simulink model "modelo_naolinear"  (R2023a)
settings: Solver=ode4  StartTime=0.0  StopTime=T_sim  FixedStep=dt  RelTol=1e-3  EnableMultiTasking=on
InitFcn:
  | modelo_naolinear_params
values from neighbouring files (symbols this model uses):
  B22 = B(7:12,2:4)    [modelo_naolinear_params.m]
  dt = 1e-3    (= 0.001)    [modelo_naolinear_params.m]
  G = inv(K*B22*inv(R)*B22'-A22')*C'*Q    [modelo_naolinear_params.m]
  g = 9.8    [modelo_naolinear_params.m]
  incluir_din_motor = 1    [modelo_naolinear_params.m]
  input_selector = 1    [modelo_naolinear_params.m]
  Ir = 1e-3    (= 0.001)    [modelo_naolinear_params.m]
  Ixx = 1e-2    (= 0.01)    [modelo_naolinear_params.m]
  Iyy = 1e-2    (= 0.01)    [modelo_naolinear_params.m]
  Izz = 1.8e-2    (= 0.018)    [modelo_naolinear_params.m]
  K = are(A22, B22*inv(R)*B22',C'*Q*C)    [modelo_naolinear_params.m]
  Kd_rot = 0.01    [modelo_naolinear_params.m]
  Kd_trans = -0.13723    [modelo_naolinear_params.m]
  Ki_rot = 1.0    (= 1)    [modelo_naolinear_params.m]
  Ki_trans = 0.4    [modelo_naolinear_params.m]
  Kp = 0.9182    [modelo_naolinear_params.m]
  Kp_rot = 10    [modelo_naolinear_params.m]
  Kp_trans = 2.0968    [modelo_naolinear_params.m]
  m = 1.0428    [modelo_naolinear_params.m]
  N_rot = 100    [modelo_naolinear_params.m]
  N_trans = 5.5815    [modelo_naolinear_params.m]
  p0 = 0    [modelo_naolinear_params.m]
  phi0 = 0    [modelo_naolinear_params.m]
  psi0 = 0    [modelo_naolinear_params.m]
  q0 = 0    [modelo_naolinear_params.m]
  R = diag([r11,r22,r33])    [modelo_naolinear_params.m]
  r0 = 0    [modelo_naolinear_params.m]
  seleciona_controlador = -1    [modelo_naolinear_params.m]
  T = [kf,kf,kf,kf ... (first row only)    [modelo_naolinear_params.m]
  T_inv = T^(-1)    [modelo_naolinear_params.m]
  tau_a = 0.0569    [modelo_naolinear_params.m]
  tau_s = 0.045    [modelo_naolinear_params.m]
  theta0 = 0    [modelo_naolinear_params.m]
  vx0 = 0    [modelo_naolinear_params.m]
  vy0 = 0    [modelo_naolinear_params.m]
  vz0 = 0    [modelo_naolinear_params.m]
  x0 = 0    [modelo_naolinear_params.m]
  y0 = 0    [modelo_naolinear_params.m]
  z0 = 1    [modelo_naolinear_params.m]
undefined symbols (not defined in the file or in its folder): sigbuilder_block
libraries: slpidlib x6, aerolibanimutils x2, sim3dlib x1, uavsim3dlib x1
(part 1 of 2)
settings Model: Creator=dferr  LastModifiedBy=dferr
settings SolverCC: AlgebraicLoopSolver=TrustRegion  AutoInsertRateTranBlk=on
settings DataIOCC: LimitDataPoints=on  SaveFormat=Array  ReturnWorkspaceOutputs=off
settings OptimizationCC: LifeSpan=inf
settings DebuggingCC: UnconnectedInputMsg=warning  UnconnectedOutputMsg=warning  UnconnectedLineMsg=warning  SFUnexpectedBacktrackingDiag=warning  SFNoUnconditionalDefaultTransitionDiag=warning  SFExecutionAtInitializationDiag=none  ArithmeticOperatorsInVariantConditions=warning
settings HardwareCC: ProdBitPerPointer=32  ProdBitPerSizeT=32  ProdBitPerPtrDiffT=32  ProdLargestAtomicFloat=None  ProdIntDivRoundTo=Undefined  ProdEndianess=Unspecified  ProdWordSize=32  ProdHWDeviceType=32-bit Generic
settings ModelReferenceCC: EnableRefExpFcnMdlSchedulingChecks=on  PropagateSignalLabelsOutOfModel=off
settings SFSimCC: SimUseLocalCustomCode=off  CustomCodeUndefinedFunction=UseInterfaceOnly
settings RTWCC: RTWUseLocalCustomCode=off
settings CodeAppCC: StateflowObjectComments=on
settings GRTTargetCC: TargetLangStandard=C89/C90 (ANSI)
settings ConfigComp: CovMetricSettings=dw  CovSaveCumulativeToWorkspaceVar=on  CovSaveSingleToWorkspaceVar=on  CovExternalEMLEnable=off  CovSFcnEnable=off

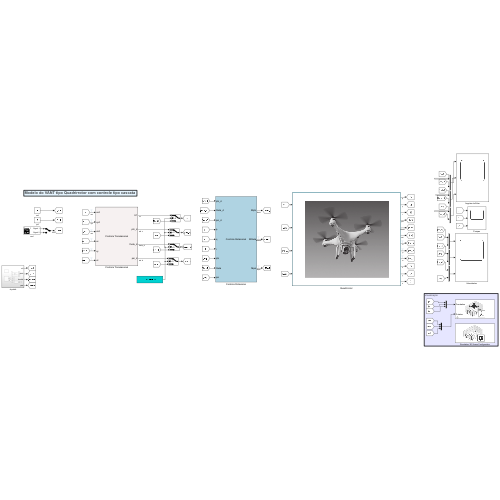
[thumbnail: Simulink's stored preview of the top-level diagram]
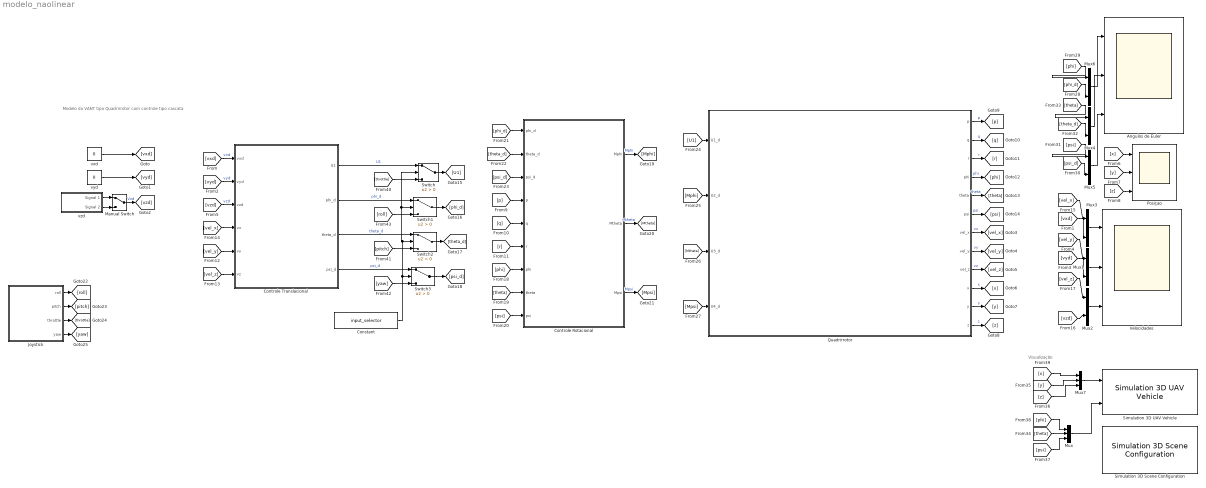
== system modelo_naolinear ==
  note: Visualização
  note: Modelo do VANT tipo Quadrirrotor com controle tipo cascata
  Angulos de Euler : Scope  NumInputPorts=3
  Constant : Constant  Value=input_selector
  Controle Rotacional : Subsystem
      mask Display:
      | disp('Controle Rotacional')
      | port_label('input',1,'phi_d')
      | port_label('input',2,'theta_d')
      | port_label('input',3,'psi_d')
      | port_label('input',4,'p')
      | port_label('input',5,'q')
      | port_label('input',6,'r')
      | port_label('input',7,'phi')
      | port_label('input',8,'theta')
      | port_label('input',9,'psi')
      | port_label('output',1,'Mphi')
      | port_label('output',2,'Mtheta')
      | port_label('output',3,'Mpsi')
  Controle Translacional : Subsystem
      mask Display:
      | disp('Controle Translacional')
      | port_label('input',1,'vxd')
      | port_label('input',2,'vyd')
      | port_label('input',3,'vzd')
      | port_label('input',4,'vx')
      | port_label('input',5,'vy')
      | port_label('input',6,'vz')
      | port_label('output',1,'U1')
      | port_label('output',2,'phi_d')
      | port_label('output',3,'theta_d')
      | port_label('output',4,'psi_d')
  Joystick : Subsystem
  Manual Switch : ManualSwitch
  Mux : Mux  Inputs=3
  Mux{1..6} (6 alike) : Mux  Inputs=2
  Mux7 : Mux  Inputs=3
  Posiçao : Scope  NumInputPorts=3
  Quadrirrotor : Subsystem
      mask init:
      | x = imread('figures/drone.png');
      | set_param(gcb,'UserDataPersistent','on');
      | ud.x = x;
      | set_param(gcb,'UserData',ud);
      mask Display:
      | disp('Quadrirrotor')
      | port_label('input',1,'U1')
      | port_label('input',2,'U2')
      | port_label('input',3,'U3')
      | port_label('input',4,'U4')
      | port_label('output',1,'p')
      | port_label('output',2,'q')
      | port_label('output',3,'r')
      | port_label('output',4,'phi')
      | port_label('output',5,'theta')
      | port_label('output',6,'psi')
      | port_label('output',7,'vel_x')
      | port_label('output',8,'vel_y')
      | port_label('output',9,'vel_z')
      | port_label('output',10,'x')
      | port_label('output',11,'y')
      | port_label('output',12,'z')
      | ud = get_param(gcb,'UserData')
      | image(ud.x)
  Simulation 3D Scene Configuration : sim3dlib/Simulation 3D Scene Configuration  Priority=0  ProjectFormat=Default Scenes  SceneDesc=US city block  ProjectName=C:\Program Files\MATLAB\R2023a\toolbox\shared\sim3d_projects\automotive_project\UE4\WindowsNoEditor\VehicleSimulation.exe  ScenePath=/Game/Maps/USCityBlock  EnableOpenDRIVE=off  vehTagList={'Scene Origin';'SimulinkVehicle1'}  vehTag=SimulinkVehicle1  OpVisEn=off  Ts=0.01  EnableWindow=on  EnableRemoteAccess=off  EnableWeather=off  SunAltitude=40.0  SunAzimuth=90  clouds=10  wind=1  fog=0  rain=0  EnableGeospatial=off  OriginHeight=200  OriginLatitude=40.744652  OriginLongitude=-73.988864  MapStyle=Aerial  AdditionalAssetIDs=[]  UseAdvancedSunSky=off  SolarTime=11  TimeZone=-5  Day=21  Month=9  Year=2022  UseDaylightSavingTime=on  DSTStartDay=10  DSTStartMonth=3  DSTEndDay=3  DSTEndMonth=11  DSTSwitchHour=2  sensorTag=MainCamera1  sensorId=1  sensorName=MainCamera1  mountLoc=Origin  offsetFlag=off  tmountOffset=[0, 0, 0]  rmountOffset=[0, 0, 0]  HorizontalResolution=1280  VerticalResolution=720  HorizontalFOV=60  mountPoint=[0 0 0]  mountOrientation=[0 0 0]  aMode=4  ID=1  GeoTrigger=Inactive
      settings: LibrarySourceBlock=uavsim3dlib/Simulation 3D Scene Configuration
  Simulation 3D UAV Vehicle : uavsim3dlib/Simulation 3D UAV Vehicle  Priority=-1  Mesh=Quadrotor  MeshPath=/MathWorksUAVContent/UAVs/HexaRotorUAV/HexaRotor.HexaRotor  Color=Black  ActorName=SimulinkVehicle1  UseGlobalFrame=off  InitialTranslation=[0 0 0]  InitialRotation=[0 0 0]  InitialTranslationGlobal=[0 0 0]  InitialGeoOrigin=[0 0 0]  InitialRotationGlobal=[0 0 0]  SampleTime=0.01  ActorType=SimulinkVehicle  ID=1  aMode=4  ActorTag=SimulinkVehicle1
  Switch{,1..3} (4 alike) : Switch  Criteria=u2 > Threshold  SaturateOnIntegerOverflow=off  InputSameDT=off
  Velocidades : Scope  NumInputPorts=3
  vxd : Constant  Value=0
  vyd : Constant  Value=0
  vzd : Subsystem  Tag=STV Subsys  LoadFcn=sigbuilder_block('load',[1045.5 36.75 386.25 279 ]);  StartFcn=sigbuilder_block('start');  StopFcn=sigbuilder_block('stop');  ClipboardFcn=sigbuilder_block('clipboard');  mask(Sigbuilder block){tuvar=[0:10]}
      mask help: Simulink:masks:SigbuilderBlock_MD
      mask init:
      | if ~strcmp(get_param(bdroot(gcbh),'SimulationStatus'),'stopped') tuvar = sigbuilder_block('maskInit'); end
      settings: CopyFcn=sigbuilder_block('copy');  DeleteFcn=sigbuilder_block('delete');  ModelCloseFcn=sigbuilder_block('modelClose');  PreSaveFcn=sigbuilder_block('preSave');  PauseFcn=sigbuilder_block('pause');  ContinueFcn=sigbuilder_block('continue');  NameChangeFcn=sigbuilder_block('namechange');  OpenFcn=sigbuilder_block('open',[437.6 199.2 550.4 400 ]);  CloseFcn=sigbuilder_block('close');
      mask Display: plot(0, 0, 100, 100,[2, 2, 32, 32, 2], [68, 8, 8, 68, 68],[32, 2], [38, 38], [32, 19, 2],[53, 60, 44], [32, 17, 17, 2],[16, 16, 31, 31]);txt = getActiveGroup(gcbh);text(2, 100, txt,'verticalAlignment', 'top');
  wires:
  Mux3/1 -> Velocidades/1
  vzd/1(Signal 1) -> Manual Switch/1
  vzd/2(Signal 2) -> Manual Switch/2
  vxd/1 -> [vxd]
  [vxd] -> Controle Translacional/1(vxd)  "vxd"
  [vxd] -> Mux3/2
  vyd/1 -> [vyd]
  [vyd] -> Controle Translacional/2(vyd)  "vyd"
  Manual Switch/1 -> [vzd]  "Vzd"
  [vzd] -> Controle Translacional/3(vzd)  "vzd"
  [x] -> Posiçao/1
  [y] -> Posiçao/2
  [z] -> Posiçao/3
  [vel_x] -> Controle Translacional/4(vx)
  [vel_y] -> Controle Translacional/5(vy)
  [vel_z] -> Controle Translacional/6(vz)
  [vel_x] -> Mux3/1
  [vel_y] -> Mux1/1
  [vyd] -> Mux1/2
  Mux1/1 -> Velocidades/2
  Mux2/1 -> Velocidades/3
  [vzd] -> Mux2/2
  [vel_z] -> Mux2/1
  Quadrirrotor/7(vel_x) -> [vel_x]  "vx"
  Quadrirrotor/8(vel_y) -> [vel_y]  "vy"
  Quadrirrotor/9(vel_z) -> [vel_z]  "vz"
  Quadrirrotor/10(x) -> [x]  "x"
  Quadrirrotor/11(y) -> [y]  "y"
  Quadrirrotor/12(z) -> [z]  "z"
  Quadrirrotor/1(p) -> [p]  "p"
  Quadrirrotor/3(r) -> [r]  "r"
  Quadrirrotor/4(phi) -> [phi]  "phi"
  Quadrirrotor/5(theta) -> [theta]  "theta"
  Quadrirrotor/6(psi) -> [psi]  "psi"
  [p] -> Controle Rotacional/4(p)
  [q] -> Controle Rotacional/5(q)
  [r] -> Controle Rotacional/6(r)
  [phi] -> Controle Rotacional/7(phi)
  [theta] -> Controle Rotacional/8(theta)
  [psi] -> Controle Rotacional/9(psi)
  [phi_d] -> Controle Rotacional/1(phi_d)
  [theta_d] -> Controle Rotacional/2(theta_d)
  [psi_d] -> Controle Rotacional/3(psi_d)
  [U1] -> Quadrirrotor/1(U1_d)
  Controle Rotacional/1(Mphi) -> [Mphi]  "Mphi"
  Controle Rotacional/2(Mtheta) -> [Mtheta]  "Mtheta"
  Controle Rotacional/3(Mpsi) -> [Mpsi]  "Mpsi"
  [Mpsi] -> Quadrirrotor/4(U4_d)
  [psi] -> Mux5/1
  [psi_d] -> Mux5/2
  Mux5/1 -> Angulos de Euler/3
  Mux4/1 -> Angulos de Euler/2
  [theta_d] -> Mux4/2
  [theta] -> Mux4/1
  [phi] -> Mux6/1
  [phi_d] -> Mux6/2
  Mux6/1 -> Angulos de Euler/1
  [Mphi] -> Quadrirrotor/2(U2_d)
  [Mtheta] -> Quadrirrotor/3(U3_d)
  Quadrirrotor/2(q) -> [q]  "q"
  Joystick/1(roll) -> [roll]
  Joystick/2(pitch) -> [pitch]
  Joystick/3(throttle) -> [throttle]
  Joystick/4(yaw) -> [yaw]
  Switch/1 -> [U1]
  Switch1/1 -> [phi_d]
  Switch2/1 -> [theta_d]
  Switch3/1 -> [psi_d]
  Controle Translacional/1(U1) -> Switch/1  "U1"
  Controle Translacional/2(phi_d) -> Switch1/1  "phi_d"
  Controle Translacional/3(theta_d) -> Switch2/1  "theta_d"
  Controle Translacional/4(psi_d) -> Switch3/1  "psi_d"
  [throttle] -> Switch/3
  [pitch] -> Switch2/3
  [yaw] -> Switch3/3
  [roll] -> Switch1/3
  Constant/1 -> Switch3/2, Switch2/2, Switch/2, Switch1/2
  [z] -> Mux7/3
  [x] -> Mux7/1
  [psi] -> Mux/3
  [y] -> Mux7/2
  Mux/1 -> Simulation 3D UAV Vehicle/2
  [phi] -> Mux/1
  [theta] -> Mux/2
  Mux7/1 -> Simulation 3D UAV Vehicle/1

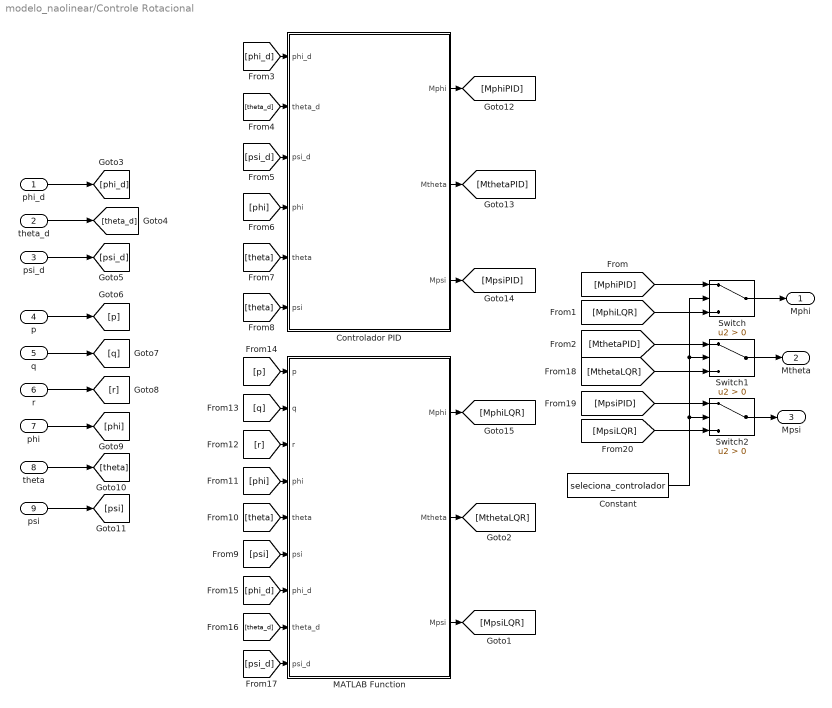
== system modelo_naolinear/Controle Rotacional ==
  phi_d : Inport
  theta_d : Inport  Port=2
  psi_d : Inport  Port=3
  p : Inport  Port=4
  q : Inport  Port=5
  r : Inport  Port=6
  phi : Inport  Port=7
  theta : Inport  Port=8
  psi : Inport  Port=9
  Constant : Constant  Value=seleciona_controlador
  Controlador PID : Subsystem
      mask Display:
      | disp('Controlador PID')
      | port_label('input',1,'phi_d')
      | port_label('input',2,'theta_d')
      | port_label('input',3,'psi_d')
      | port_label('input',4,'phi')
      | port_label('input',5,'theta')
      | port_label('input',6,'psi')
      | port_label('output',1,'Mphi')
      | port_label('output',2,'Mtheta')
      | port_label('output',3,'Mpsi')
  MATLAB Function : MATLAB Function  TreatAsAtomicUnit=on
  Switch : Switch  Criteria=u2 > Threshold  SaturateOnIntegerOverflow=off  InputSameDT=off
  Switch1 : Switch  Criteria=u2 > Threshold  SaturateOnIntegerOverflow=off  InputSameDT=off
  Switch2 : Switch  Criteria=u2 > Threshold  SaturateOnIntegerOverflow=off  InputSameDT=off
  Mphi : Outport  VectorParamsAs1DForOutWhenUnconnected=off
  Mtheta : Outport  Port=2  VectorParamsAs1DForOutWhenUnconnected=off
  Mpsi : Outport  Port=3  VectorParamsAs1DForOutWhenUnconnected=off
  wires:
  Switch/1 -> Mphi/1
  Switch1/1 -> Mtheta/1
  Switch2/1 -> Mpsi/1
  phi_d/1 -> [phi_d]
  theta_d/1 -> [theta_d]
  psi_d/1 -> [psi_d]
  p/1 -> [p]
  q/1 -> [q]
  r/1 -> [r]
  phi/1 -> [phi]
  theta/1 -> [theta]
  psi/1 -> [psi]
  [phi_d] -> Controlador PID/1(phi_d)
  [theta_d] -> Controlador PID/2(theta_d)
  [psi_d] -> Controlador PID/3(psi_d)
  [phi] -> Controlador PID/4(phi)
  [theta] -> Controlador PID/5(theta)
  [theta] -> Controlador PID/6(psi)
  Controlador PID/3(Mpsi) -> [MpsiPID]
  Controlador PID/2(Mtheta) -> [MthetaPID]
  Controlador PID/1(Mphi) -> [MphiPID]
  [psi] -> MATLAB Function/6(psi)
  [theta] -> MATLAB Function/5(theta)
  [phi] -> MATLAB Function/4(phi)
  [r] -> MATLAB Function/3(r)
  [q] -> MATLAB Function/2(q)
  [p] -> MATLAB Function/1(p)
  [phi_d] -> MATLAB Function/7(phi_d)
  [theta_d] -> MATLAB Function/8(theta_d)
  [psi_d] -> MATLAB Function/9(psi_d)
  MATLAB Function/1(Mphi) -> [MphiLQR]
  MATLAB Function/2(Mtheta) -> [MthetaLQR]
  MATLAB Function/3(Mpsi) -> [MpsiLQR]
  [MphiPID] -> Switch/1
  [MphiLQR] -> Switch/3
  [MthetaPID] -> Switch1/1
  [MthetaLQR] -> Switch1/3
  [MpsiPID] -> Switch2/1
  [MpsiLQR] -> Switch2/3
  Constant/1 -> Switch2/2, Switch1/2, Switch/2

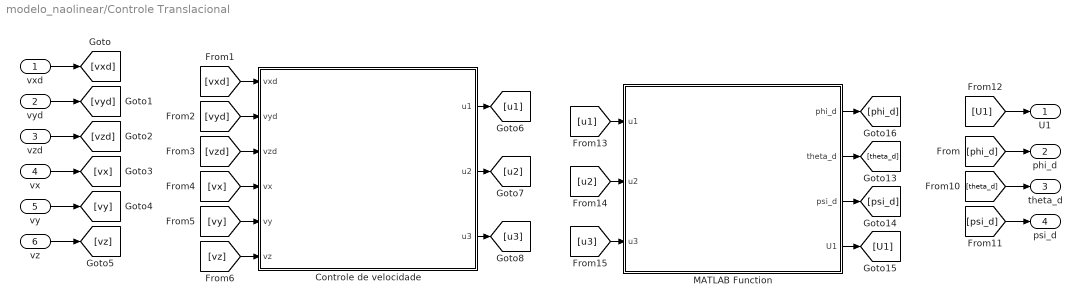
== system modelo_naolinear/Controle Translacional ==
  vxd : Inport
  vyd : Inport  Port=2
  vzd : Inport  Port=3
  vx : Inport  Port=4
  vy : Inport  Port=5
  vz : Inport  Port=6
  Controle de velocidade : Subsystem
      mask Display:
      | disp('Controle de Velocidade PID')
      | port_label('input',1,'vxd')
      | port_label('input',2,'vyd')
      | port_label('input',3,'vzd')
      | port_label('input',4,'vx')
      | port_label('input',5,'vy')
      | port_label('input',6,'vz')
      | 
      | port_label('output',1,'u1')
      | port_label('output',2,'u2')
      | port_label('output',3,'u3')
  MATLAB Function : MATLAB Function  TreatAsAtomicUnit=on
  U1 : Outport  VectorParamsAs1DForOutWhenUnconnected=off
  phi_d : Outport  Port=2  VectorParamsAs1DForOutWhenUnconnected=off
  theta_d : Outport  Port=3  VectorParamsAs1DForOutWhenUnconnected=off
  psi_d : Outport  Port=4  VectorParamsAs1DForOutWhenUnconnected=off
  wires:
  vxd/1 -> [vxd]
  vyd/1 -> [vyd]
  vzd/1 -> [vzd]
  vx/1 -> [vx]
  vy/1 -> [vy]
  vz/1 -> [vz]
  [vxd] -> Controle de velocidade/1(vxd)
  [vyd] -> Controle de velocidade/2(vyd)
  [vzd] -> Controle de velocidade/3(vzd)
  [vx] -> Controle de velocidade/4(vx)
  [vy] -> Controle de velocidade/5("vy ")
  [vz] -> Controle de velocidade/6(vz)
  Controle de velocidade/1(u1) -> [u1]
  Controle de velocidade/2(u2) -> [u2]
  Controle de velocidade/3(u3) -> [u3]
  [phi_d] -> phi_d/1
  [theta_d] -> theta_d/1
  [psi_d] -> psi_d/1
  [U1] -> U1/1
  [u1] -> MATLAB Function/1(u1)
  [u2] -> MATLAB Function/2(u2)
  [u3] -> MATLAB Function/3(u3)
  MATLAB Function/1(phi_d) -> [phi_d]
  MATLAB Function/2(theta_d) -> [theta_d]
  MATLAB Function/3(psi_d) -> [psi_d]
  MATLAB Function/4(U1) -> [U1]

== system modelo_naolinear/Joystick ==
  Demux : Demux  Outputs=6
  Fcn : Fcn  Expr=(u(1)-0.5)*2
  Gain : Gain  Gain=-1
  Pilot Joystick1 : aerolibanimutils/Pilot Joystick  JoystickID=None  OutputConfiguration=AllOutputs  SampleTime=dt
  Simulation Pace : aerolibanimutils/Simulation Pace  SimulationPace=1  SleepMode=Auto  OutputPaceError=off  SampleTime=dt
  Terminator{,1..3} (4 alike) : Terminator
  roll : Outport
  pitch : Outport  Port=2
  throttle : Outport  Port=3
  yaw : Outport  Port=4
  wires:
  Demux/4 -> Terminator2/1
  Gain/1 -> pitch/1  "pitch"
  Pilot Joystick1/3 -> Terminator1/1
  Pilot Joystick1/1 -> Demux/1
  Demux/6 -> Fcn/1
  Pilot Joystick1/2 -> Terminator/1
  Demux/2 -> Gain/1
  Demux/1 -> roll/1  "roll"
  Demux/5 -> Terminator3/1
  Demux/3 -> throttle/1  "throttle"
  Fcn/1 -> yaw/1  "yaw"

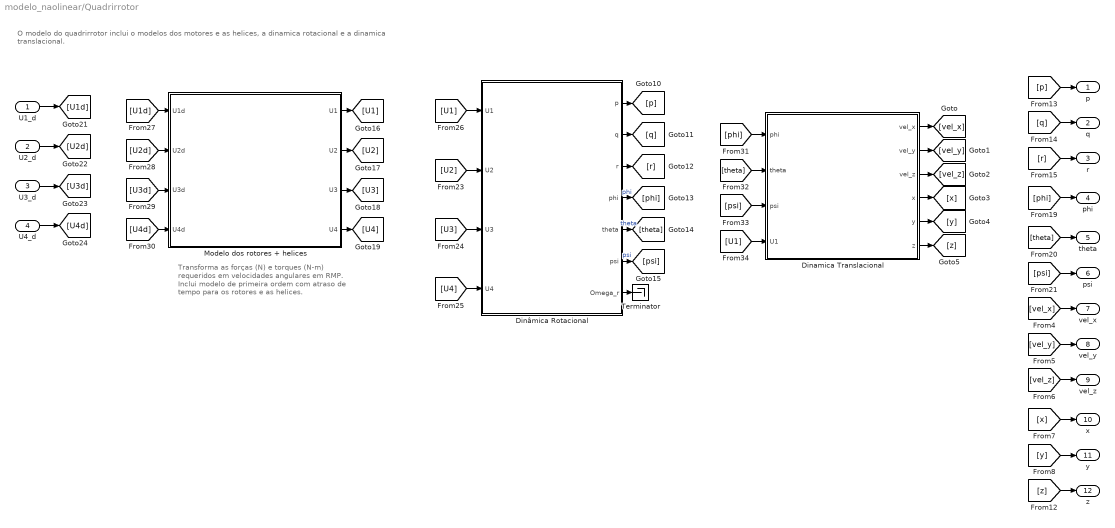
== system modelo_naolinear/Quadrirrotor ==
  note: O modelo do quadrirrotor inclui o modelos dos motores e as helices,
      | a dinamica rotacional e a dinamica translacional.
  note: Transforma as forças (N) e torques (N-m)
      | requeridos em velocidades angulares
      | em RMP. Inclui modelo de primeira
      | ordem com atraso de tempo para
      | os rotores e as helices.
  U1_d : Inport
  U2_d : Inport  Port=2
  U3_d : Inport  Port=3
  U4_d : Inport  Port=4
  Dinamica Translacional : Subsystem
  Dinâmica Rotacional : Subsystem
      mask Display:
      | disp('Dinâmica Rotacional')
      | port_label('input',1,'U1')
      | port_label('input',2,'U2')
      | port_label('input',3,'U3')
      | port_label('input',4,'U4')
      | port_label('output',1,'p')
      | port_label('output',2,'q')
      | port_label('output',3,'r')
      | port_label('output',4,'phi')
      | port_label('output',5,'theta')
      | port_label('output',6,'psi')
      | port_label('output',7,'Omega_r')
  "Modelo dos rotores + helices " : Subsystem
      mask init:
      | x = imread('figures/brushless_propellers.png');
      | set_param(gcb,'UserDataPersistent','on');
      | ud.x = x;
      | set_param(gcb,'UserData',ud);
      mask Display:
      | disp('Dinâmica Motores')
      | port_label('input',1,'U1d')
      | port_label('input',2,'U2d')
      | port_label('input',3,'U3d')
      | port_label('input',4,'U4d')
      | port_label('output',1,'U1')
      | port_label('output',2,'U2')
      | port_label('output',3,'U3')
      | port_label('output',4,'U4')
      | image(ud.x)
  Terminator : Terminator
  p : Outport  VectorParamsAs1DForOutWhenUnconnected=off
  q : Outport  Port=2  VectorParamsAs1DForOutWhenUnconnected=off
  r : Outport  Port=3  VectorParamsAs1DForOutWhenUnconnected=off
  phi : Outport  Port=4  VectorParamsAs1DForOutWhenUnconnected=off
  theta : Outport  Port=5  VectorParamsAs1DForOutWhenUnconnected=off
  psi : Outport  Port=6  VectorParamsAs1DForOutWhenUnconnected=off
  vel_x : Outport  Port=7  VectorParamsAs1DForOutWhenUnconnected=off
  vel_y : Outport  Port=8  VectorParamsAs1DForOutWhenUnconnected=off
  vel_z : Outport  Port=9  VectorParamsAs1DForOutWhenUnconnected=off
  x : Outport  Port=10  VectorParamsAs1DForOutWhenUnconnected=off
  y : Outport  Port=11  VectorParamsAs1DForOutWhenUnconnected=off
  z : Outport  Port=12  VectorParamsAs1DForOutWhenUnconnected=off
  wires:
  [vel_x] -> vel_x/1
  [vel_y] -> vel_y/1
  [vel_z] -> vel_z/1
  [z] -> z/1
  [y] -> y/1
  [x] -> x/1
  Dinâmica Rotacional/3(r) -> [r]
  Dinâmica Rotacional/2(q) -> [q]
  Dinâmica Rotacional/1(p) -> [p]
  [p] -> p/1
  [q] -> q/1
  [r] -> r/1
  Dinâmica Rotacional/5(theta) -> [theta]  "theta"
  Dinâmica Rotacional/6(psi) -> [psi]  "psi"
  [phi] -> phi/1
  [theta] -> theta/1
  [psi] -> psi/1
  "Modelo dos rotores + helices "/1(U1) -> [U1]
  "Modelo dos rotores + helices "/2(U2) -> [U2]
  "Modelo dos rotores + helices "/3(U3) -> [U3]
  "Modelo dos rotores + helices "/4(U4) -> [U4]
  [U1d] -> "Modelo dos rotores + helices "/1(U1d)
  [U2d] -> "Modelo dos rotores + helices "/2(U2d)
  [U3d] -> "Modelo dos rotores + helices "/3(U3d)
  [U4d] -> "Modelo dos rotores + helices "/4(U4d)
  U1_d/1 -> [U1d]
  U2_d/1 -> [U2d]
  U3_d/1 -> [U3d]
  U4_d/1 -> [U4d]
  [U4] -> Dinâmica Rotacional/4(U4)
  [U3] -> Dinâmica Rotacional/3(U3)
  [U2] -> Dinâmica Rotacional/2(U2)
  [U1] -> Dinâmica Rotacional/1(U1)
  Dinâmica Rotacional/7(Omega_r) -> Terminator/1
  Dinâmica Rotacional/4(phi) -> [phi]  "phi"
  [phi] -> Dinamica Translacional/1(phi)
  [theta] -> Dinamica Translacional/2(theta)
  [psi] -> Dinamica Translacional/3(psi)
  [U1] -> Dinamica Translacional/4(U1)
  Dinamica Translacional/1(vel_x) -> [vel_x]
  Dinamica Translacional/2(vel_y) -> [vel_y]
  Dinamica Translacional/3(vel_z) -> [vel_z]
  Dinamica Translacional/4(x) -> [x]
  Dinamica Translacional/5(y) -> [y]
  Dinamica Translacional/6(z) -> [z]

== system modelo_naolinear/vzd ==
  Demux : Demux  Tag=STV Demux  Outputs=2
      settings: DisplayOption=none
  FromWs : FromWorkspace  Tag=STV FromWs  VariableName=tuvar
      SigBuilderData=|
      | common.afterFinalStr = 'Extrapolate'
      | common.sampleTime = 0
      | dataSet.timeRange = [0 20]
      | dataSet.name = 'Group 1'
      | sbobj.Groups.Name = 'Group 1'
      | sbobj.Groups.Signals(1).Name = 'Signal 1'
      | sbobj.Groups.Signals(1).XData = [0 2.05 4.75 8 18 20]
      | sbobj.Groups.Signals(1).YData = [-0.2 4.2 10 10 0 0]
      | sbobj.Groups.Signals(2).Name = 'Signal 2'
      | sbobj.Groups.Signals(2).XData = [0 5 5 20]
      | sbobj.Groups.Signals(2).YData = [0 0 1 1]
      | sbobj.Groups.TimeRange = [0 20]
  Signal 1 : Outport  Tag=STV Outport  VectorParamsAs1DForOutWhenUnconnected=off
  Signal 2 : Outport  Tag=STV Outport  Port=2  VectorParamsAs1DForOutWhenUnconnected=off
  wires:
  FromWs/1 -> Demux/1
  Demux/1 -> Signal 1/1
  Demux/2 -> Signal 2/1

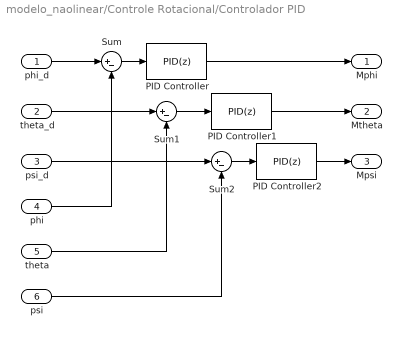
== system modelo_naolinear/Controle Rotacional/Controlador PID ==
  phi_d : Inport
  theta_d : Inport  Port=2
  psi_d : Inport  Port=3
  phi : Inport  Port=4
  theta : Inport  Port=5
  psi : Inport  Port=6
  PID Controller : slpidlib/PID Controller  Controller=PID  Form=Parallel  TimeDomain=Discrete-time  UseExternalTs=off  SampleTime=-1  IntegratorMethod=Forward Euler  FilterMethod=Forward Euler  ControllerParametersSource=internal  P=Kp_rot  I=Ki_rot  UseKiTs=off  D=Kd_rot  N=N_rot  UseFilter=on  TunerSelectOption=Transfer Function Based (PID Tuner App)  InitialConditionSource=internal  InitialConditionForIntegrator=0  InitialConditionForFilter=0  DifferentiatorICPrevScaledInput=0  ExternalReset=none  TrackingMode=off  Kt=1  LimitOutput=on  SatLimitsSource=internal  UpperSaturationLimit=inf  LowerSaturationLimit=-inf  LinearizeAsGain=on  AntiWindupMode=clamping  Kb=1  LimitIntegrator=off  UpperIntegratorSaturationLimit=inf  LowerIntegratorSaturationLimit=-inf  POutMin=[]  POutMax=[]  IOutMin=[]  IOutMax=[]  DOutMin=[]  DOutMax=[]  NOutMin=[]  NOutMax=[]  SumOutMin=[]  SumOutMax=[]  SaturationOutMin=[]  SaturationOutMax=[]  PParamMin=[]  PParamMax=[]  IParamMin=[]  IParamMax=[]  DParamMin=[]  DParamMax=[]  NParamMin=[]  NParamMax=[]  KbParamMin=[]  KbParamMax=[]  KtParamMin=[]  KtParamMax=[]  KbOutMin=[]  KbOutMax=[]  KtOutMin=[]  KtOutMax=[]  IntegratorOutMin=[]  IntegratorOutMax=[]  FilterOutMin=[]  FilterOutMax=[]  SumI1OutMin=[]  SumI1OutMax=[]  SumI2OutMin=[]  SumI2OutMax=[]  SumI3OutMin=[]  SumI3OutMax=[]  SumI4OutMin=[]  SumI4OutMax=[]  SumDOutMin=[]  SumDOutMax=[]  DifferentiatorOutMin=[]  DifferentiatorOutMax=[]  FilterDiffOutCoefMin=[]  FilterDiffOutCoefMax=[]  ReciprocalOutMin=[]  ReciprocalOutMax=[]  SumDenOutMin=[]  SumDenOutMax=[]  SumNumOutMin=[]  SumNumOutMax=[]  DivideOutMin=[]  DivideOutMax=[]  UdiffTsProdOutMin=[]  UdiffTsProdOutMax=[]  NTsProdOutMin=[]  NTsProdOutMax=[]  UintegralTsProdOutMin=[]  UintegralTsProdOutMax=[]  UngainTsProdOutMin=[]  UngainTsProdOutMax=[]  ClampingZeroOutDataTypeStr=Inherit: Inherit via back propagation  ClampingZeroOutMin=[]  ClampingZeroOutMax=[]  FilterDenConstantOutDataTypeStr=Inherit: Inherit via back propagation  FilterDenConstantOutMin=[]  FilterDenConstantOutMax=[]  PCopyOutDataTypeStr=Inherit: Inherit via back propagation  PCopyOutMin=[]  PCopyOutMax=[]  NCopyOutDataTypeStr=Inherit: Inherit via back propagation  NCopyOutMin=[]  NCopyOutMax=[]  IntegratorContinuousStateAttributes=''  IntegratorStateMustResolveToSignalObject=off  IntegratorRTWStateStorageClass=Auto  FilterContinuousStateAttributes=''  FilterStateMustResolveToSignalObject=off  FilterRTWStateStorageClass=Auto  ParallelPVariant=InternalParameters  IdealPVariant=Disabled  IVariant=InternalParameters  DVariant=InternalParameters  IntegratorVariant=Discrete  SatVariant=Enabled  AWVariant=DiscClampingParallel  PCopyVariant=Disabled  TRVariant=Disabled  FdbkBlocksVariant=Forward  IdealPFdbkVariant=Disabled  SatFdbkVariant=Disabled  DerivativeFilterVariant=ForwardEulerFilter  NVariant=InternalParameters  NCopyVariant=Disabled  FilterICVariant=InternalICsFilter  IntegratorICVariant=InternalICs  ExternalResetVariant=Disabled  TRSumVariant=Passthrough  SumFdbkVariant=Disabled  SumVariant=Sum_PID  TsampFilterVariant=InternalTs  TsampNgainVariant=Passthrough  TsampIntegralVariant=Disabled_SignalSpec  ExternalSaturationOutDataTypeStr=Inherit: Same as second input